simulink model "HvacMdlAirFlowDistbnFrnt"  (R2022b)
settings: Solver=FixedStepDiscrete  StartTime=0.0  StopTime=10.0  FixedStep=0.2  RelTol=1e-3
(part 1 of 2)
settings Model: Creator=blue.ge  LastModifiedBy=blue.ge
settings DataIOCC: SaveFormat=StructureWithTime
settings OptimizationCC: DefaultParameterBehavior=Inlined  EnhancedBackFolding=on  PassReuseOutputArgsAs=Individual arguments  ZeroExternalMemoryAtStartup=off  ZeroInternalMemoryAtStartup=off  OptimizeBlockOrder=speed  DifferentSizesBufferReuse=on  OptimizationCustomize=off  EfficientTunableParamExpr=on
settings DebuggingCC: ParameterTunabilityLossMsg=error
settings ModelReferenceCC: ModelReferenceNumInstancesAllowed=Single
settings SFSimCC: MATLABDynamicMemAlloc=off
settings RTWCC: Description=AUTOSAR  SystemTargetFile=autosar.tlc  GenCodeOnly=on  TemplateMakefile=ert_default_tmf
settings CodeAppCC: ForceParamTrailComments=on  IgnoreCustomStorageClasses=off  OperatorAnnotations=on  SimulinkDataObjDesc=on  SFDataObjDesc=on  InsertBlockDesc=on
settings STFCustomTargetCC: TargetLangStandard=C99 (ISO)  MultiwordLength=256  ParMdlRefBuildCompliant=on  CompOptLevelCompliant=on  IncludeMdlTerminateFcn=off  CombineOutputUpdateFcns=on  SuppressErrorStatus=on  ERTCustomFileBanners=on  SupportNonFinite=off  SupportComplex=off  SupportContinuousTime=off  SupportNonInlinedSFcns=off  RemoveResetFunc=on  MATLABClassNameForMDSCustomization=Simulink.SoftwareTarget.ERTCustomization  AutosarCompliant=on  UseToolchainInfoCompliant=on  LookupTableObjectStructAxisOrder=2,1,3,4,...  LUTObjectStructOrderEvenSpacing=Size,Table,Breakpoints  SystemTargetFile=autosar.tlc
settings ERTTargetCC: TargetLangStandard=C99 (ISO)  ConcurrentExecutionCompliant=off  IncludeMdlTerminateFcn=off  SuppressErrorStatus=on  SupportNonFinite=off  SupportComplex=off  ModelStepFunctionPrototypeControlCompliant=off  CPPClassGenCompliant=off  AutosarCompliant=on  LookupTableObjectStructAxisOrder=2,1,3,4,...  LUTObjectStructOrderEvenSpacing=Size,Table,Breakpoints  InlinedParameterPlacement=NonHierarchical  ERTCustomFileTemplate=example_file_process.tlc

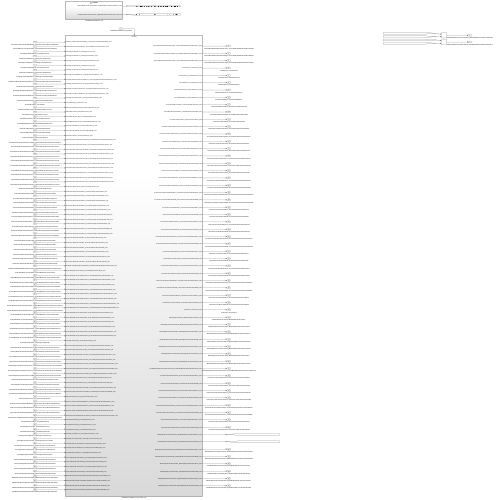
[thumbnail: Simulink's stored preview of the top-level diagram]
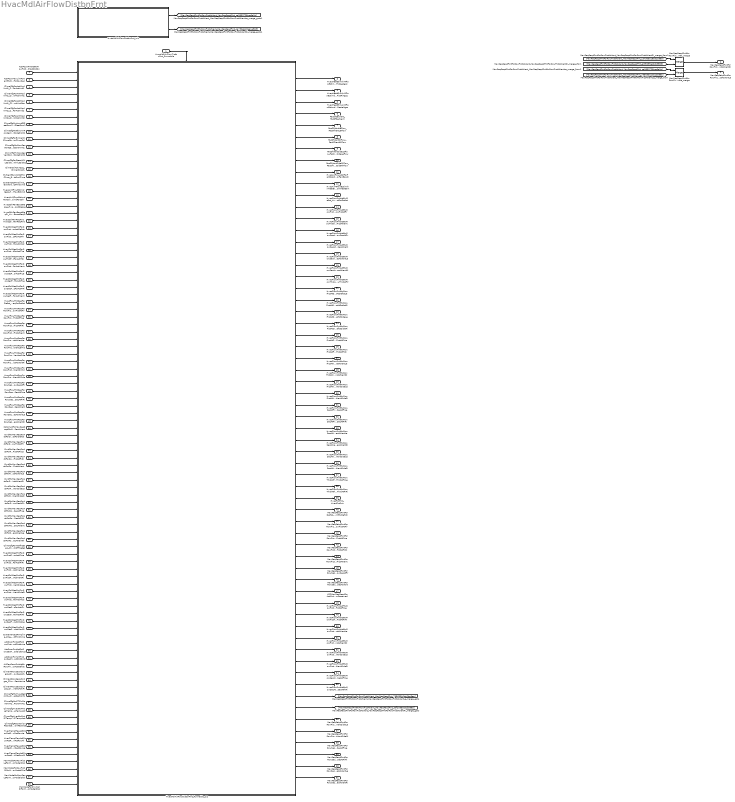
== system HvacMdlAirFlowDistbnFrnt ==
  HvacMdlAirFlowDistbnFrnt_Runnable : Inport  SampleTime=0.2  OutputFunctionCall=on
  ActrPosnFrntActForRowFirstModDistbn_ActrPosnFrntActForRowFirstModDistbn : Inport  Port=2  OutMin=0  OutMax=100  OutDataTypeStr=Perc9  PortDimensions=1
  ActrPosnMaxLimForVentForRowSec_ActrPosnMaxLimForVentForRowSec : Inport  Port=3  OutDataTypeStr=Perc30  PortDimensions=1
  ClimaCfgForActrModVrnt1_ClimaCfgForActrInd1 : Inport  Port=4  OutDataTypeStr=Enum: ClimaCfgForActrInd1  PortDimensions=1
  ClimaCfgForActrModVrnt1_ClimaCfgForActrModVrnt1 : Inport  Port=5  OutDataTypeStr=Enum: ClimaCfgForActrModVrnt1  PortDimensions=1
  ClimaCfgForActrModVrnt1_ClimaCfgForActrModVrntCen : Inport  Port=6  OutDataTypeStr=Enum: ClimaCfgForActrModVrntCen  PortDimensions=1
  ClimaCfgForActrModVrnt2_ClimaCfgForActrInd2 : Inport  Port=7  OutDataTypeStr=Enum: ClimaCfgForActrInd2  PortDimensions=1
  ClimaCfgForActrModVrnt2_ClimaCfgForActrModVrnt2 : Inport  Port=8  OutDataTypeStr=Enum: ClimaCfgForActrModVrnt2  PortDimensions=1
  ClimaCfgForAmntOfSeatRow1_ClimaCfgForAmntOfSeatRow1 : Inport  Port=9  OutDataTypeStr=Enum: ClimaCfgForAmntOfSeatRow1  PortDimensions=1
  ClimaCfgForBlowrInRowSecVentSidePrsnt1_ClimaCfgForBlowrInRowSecVentSidePrsnt1 : Inport  Port=10  OutDataTypeStr=Boolean  PortDimensions=1
  ClimaCfgForExtVentnClimaCtrl_ClimaCfgForExtVentnClimaCtrl : Inport  Port=11  OutDataTypeStr=Enum: CarCfgForExtVentnClimaCtrl  PortDimensions=1
  ClimaCfgForRowSecVentCentrVrnt1_ClimaCfgForRowSecVentCentrVrnt1 : Inport  Port=12  OutDataTypeStr=Enum: ClimaCfgForRowSecVentCentrVrnt1  PortDimensions=1
  ClimaCfgForRowSecVentSidePrsnt1_ClimaCfgForRowSecVentSidePrsnt1 : Inport  Port=13  OutDataTypeStr=Boolean  PortDimensions=1
  ClimaCfgForSteerWhlLeSide1_ClimaCfgForSteerWhlLeSide1 : Inport  Port=14  OutDataTypeStr=Boolean  PortDimensions=1
  ClimaCtrlActvNgcc_ClimaCtrlActv : Inport  Port=15  OutDataTypeStr=Boolean  PortDimensions=1
  ExtVentFanVolStsFrmClima_ExtVentFanVolStsFrmClima : Inport  Port=16  OutDataTypeStr=Enum: Level4  PortDimensions=1
  ExtVentStsFrmClima_ExtVentStsFrmClima : Inport  Port=17  OutDataTypeStr=Enum: OnOff1  PortDimensions=1
  HvacAirMFlowEstimd_Ngcc_HvacAirMFlowEstimd : Inport  Port=18  OutMin=0  OutMax=1000  OutDataTypeStr=MFlow1  PortDimensions=1
  HvacAirMFlowEstimdForCtrlr_HvacAirMFlowEstimdForCtrlr : Inport  Port=19  OutDataTypeStr=AirFlow30  PortDimensions=1
  HvacCtrlrFanBoostReqLe_HvacCtrlrFanBoostReqLe : Inport  Port=20  OutDataTypeStr=Perc30  PortDimensions=1
  HvacCtrlrFanBoostReqRi_HvacCtrlrFanBoostReqRi : Inport  Port=21  OutDataTypeStr=Perc30  PortDimensions=1
  HvacCtrlrFanReqFrnt_HvacCtrlrFanReqFrnt : Inport  Port=22  OutDataTypeStr=FanSpd1  PortDimensions=1
  HvacCtrlrReqFrntForRowFirstForActrDefrst_HvacCtrlrReqFrntForRowFirstForActrDefrst : Inport  Port=23  OutDataTypeStr=Perc30  PortDimensions=1
  HvacCtrlrReqFrntForRowFirstForActrFlr_HvacCtrlrReqFrntForRowFirstForActrFlr : Inport  Port=24  OutDataTypeStr=Perc30  PortDimensions=1
  HvacCtrlrReqFrntForRowFirstForActrMod_HvacCtrlrReqFrntForRowFirstForActrMod : Inport  Port=25  OutDataTypeStr=Perc30  PortDimensions=1
  HvacCtrlrReqFrntForRowFirstForActrTLe_HvacCtrlrReqFrntForRowFirstForActrTLe : Inport  Port=26  OutDataTypeStr=Perc30  PortDimensions=1
  HvacCtrlrReqFrntForRowFirstForActrTRi_HvacCtrlrReqFrntForRowFirstForActrTRi : Inport  Port=27  OutDataTypeStr=Perc30  PortDimensions=1
  HvacCtrlrReqFrntForRowFirstForActrVent_HvacCtrlrReqFrntForRowFirstForActrVent : Inport  Port=28  OutDataTypeStr=Perc30  PortDimensions=1
  HvacCtrlrReqFrntForRowSecForActrFlr_HvacCtrlrReqFrntForRowSecForActrFlrLe : Inport  Port=29  OutDataTypeStr=Perc30  PortDimensions=1
  HvacCtrlrReqFrntForRowSecForActrTLe_HvacCtrlrReqFrntForRowSecForActrTLe : Inport  Port=30  OutDataTypeStr=Perc30  PortDimensions=1
  HvacCtrlrReqFrntForRowSecForActrTRi_HvacCtrlrReqFrntForRowSecForActrTRi : Inport  Port=31  OutDataTypeStr=Perc30  PortDimensions=1
  HvacCtrlrReqFrntForRowSecForActrVent_HvacCtrlrReqFrntForRowSecForActrVent : Inport  Port=32  OutDataTypeStr=Perc30  PortDimensions=1
  HvacFlowFrntReqForDefrst_HvacFlowFrntReqForDefrst : Inport  Port=33  OutDataTypeStr=AirFlow30  PortDimensions=1
  HvacFlowFrntReqForRowFirstAtFlr_HvacFlowFrntReqForRowFirstAtFlr : Inport  Port=34  OutDataTypeStr=AirFlow30  PortDimensions=1
  HvacFlowFrntReqForRowFirstAtFlr_HvacFlowFrntReqForRowFirstAtFlrLe : Inport  Port=35  OutDataTypeStr=AirFlow30  PortDimensions=1
  HvacFlowFrntReqForRowFirstAtFlr_HvacFlowFrntReqForRowFirstAtFlrRi : Inport  Port=36  OutDataTypeStr=AirFlow30  PortDimensions=1
  HvacFlowFrntReqForRowFirstAtVent_HvacFlowFrntReqForRowFirstAtVent : Inport  Port=37  OutDataTypeStr=AirFlow30  PortDimensions=1
  HvacFlowFrntReqForRowFirstAtVent_HvacFlowFrntReqForRowFirstAtVentLe : Inport  Port=38  OutDataTypeStr=AirFlow30  PortDimensions=1
  HvacFlowFrntReqForRowFirstAtVent_HvacFlowFrntReqForRowFirstAtVentLeMid : Inport  Port=39  OutDataTypeStr=AirFlow30  PortDimensions=1
  HvacFlowFrntReqForRowFirstAtVent_HvacFlowFrntReqForRowFirstAtVentLeSide : Inport  Port=40  OutDataTypeStr=AirFlow30  PortDimensions=1
  HvacFlowFrntReqForRowFirstAtVent_HvacFlowFrntReqForRowFirstAtVentRi : Inport  Port=41  OutDataTypeStr=AirFlow30  PortDimensions=1
  HvacFlowFrntReqForRowFirstAtVent_HvacFlowFrntReqForRowFirstAtVentRiMid : Inport  Port=42  OutDataTypeStr=AirFlow30  PortDimensions=1
  HvacFlowFrntReqForRowFirstAtVent_HvacFlowFrntReqForRowFirstAtVentRiSide : Inport  Port=43  OutDataTypeStr=AirFlow30  PortDimensions=1
  HvacFlowFrntReqForRowSecAtFlr_HvacFlowFrntReqForRowSecAtFlr : Inport  Port=44  OutDataTypeStr=AirFlow30  PortDimensions=1
  HvacFlowFrntReqForRowSecAtFlr_HvacFlowFrntReqForRowSecAtFlrLe : Inport  Port=45  OutDataTypeStr=AirFlow30  PortDimensions=1
  HvacFlowFrntReqForRowSecAtFlr_HvacFlowFrntReqForRowSecAtFlrRi : Inport  Port=46  OutDataTypeStr=AirFlow30  PortDimensions=1
  HvacFlowFrntReqForRowSecAtVent_HvacFlowFrntReqForRowSecAtVent : Inport  Port=47  OutDataTypeStr=AirFlow30  PortDimensions=1
  HvacFlowFrntReqForRowSecAtVent_HvacFlowFrntReqForRowSecAtVentLe : Inport  Port=48  OutDataTypeStr=AirFlow30  PortDimensions=1
  HvacFlowFrntReqForRowSecAtVent_HvacFlowFrntReqForRowSecAtVentRi : Inport  Port=49  OutDataTypeStr=AirFlow30  PortDimensions=1
  NotAllwdForMaxReqReqdForRowSecAtVent_NotAllwdForMaxReqReqdForRowSecAtVent : Inport  Port=50  OutDataTypeStr=Boolean  PortDimensions=1
  OvrdForMaxReqForActrForDefrst_OvrdForMaxReqForActrForDefrst : Inport  Port=51  OutDataTypeStr=Boolean  PortDimensions=1
  OvrdForMaxReqForActrForRowFirstAtFlr_OvrdForMaxReqForActrForRowFirstAtFlr : Inport  Port=52  OutDataTypeStr=Boolean  PortDimensions=1
  OvrdForMaxReqForActrForRowFirstAtFlr_OvrdForMaxReqForActrForRowFirstAtFlrLe : Inport  Port=53  OutDataTypeStr=Boolean  PortDimensions=1
  OvrdForMaxReqForActrForRowFirstAtFlr_OvrdForMaxReqForActrForRowFirstAtFlrRi : Inport  Port=54  OutDataTypeStr=Boolean  PortDimensions=1
  OvrdForMaxReqForActrForRowFirstAtVent_OvrdForMaxReqForActrForRowFirstAtVent : Inport  Port=55  OutDataTypeStr=Boolean  PortDimensions=1
  OvrdForMaxReqForActrForRowFirstAtVent_OvrdForMaxReqForActrForRowFirstAtVentLe : Inport  Port=56  OutDataTypeStr=Boolean  PortDimensions=1
  OvrdForMaxReqForActrForRowFirstAtVent_OvrdForMaxReqForActrForRowFirstAtVentRi : Inport  Port=57  OutDataTypeStr=Boolean  PortDimensions=1
  OvrdForMaxReqForActrForRowFirstAtVent_OvrdForMaxReqForActrForRowFirstAtVentSideLe : Inport  Port=58  OutDataTypeStr=Boolean  PortDimensions=1
  OvrdForMaxReqForActrForRowFirstAtVent_OvrdForMaxReqForActrForRowFirstAtVentSideRi : Inport  Port=59  OutDataTypeStr=Boolean  PortDimensions=1
  OvrdForMaxReqForActrForRowSecAtFlr_OvrdForMaxReqForActrForRowSecAtFlr : Inport  Port=60  OutDataTypeStr=Boolean  PortDimensions=1
  OvrdForMaxReqForActrForRowSecAtFlr_OvrdForMaxReqForActrForRowSecAtFlrLe : Inport  Port=61  OutDataTypeStr=Boolean  PortDimensions=1
  OvrdForMaxReqForActrForRowSecAtFlr_OvrdForMaxReqForActrForRowSecAtFlrRi : Inport  Port=62  OutDataTypeStr=Boolean  PortDimensions=1
  OvrdForMaxReqForActrForRowSecAtVent_OvrdForMaxReqForActrForRowSecAtVent : Inport  Port=63  OutDataTypeStr=Boolean  PortDimensions=1
  OvrdForMaxReqForActrForRowSecAtVent_OvrdForMaxReqForActrForRowSecAtVentLe : Inport  Port=64  OutDataTypeStr=Boolean  PortDimensions=1
  OvrdForMaxReqForActrForRowSecAtVent_OvrdForMaxReqForActrForRowSecAtVentRi : Inport  Port=65  OutDataTypeStr=Boolean  PortDimensions=1
  ClimaCfgForNrOfTSetg1_ClimaCfgForNrOfTSetg1 : Inport  Port=66  OutDataTypeStr=Enum: ClimaCfgForNrOfTSetg1  PortDimensions=1
  HvacCtrlrReqFrntForRowFirstForActrFlr_HvacCtrlrReqFrntForRowFirstForActrFlrLe : Inport  Port=67  OutDataTypeStr=Perc30  PortDimensions=1
  HvacCtrlrReqFrntForRowFirstForActrFlr_HvacCtrlrReqFrntForRowFirstForActrFlrRi : Inport  Port=68  OutDataTypeStr=Perc30  PortDimensions=1
  HvacCtrlrReqFrntForRowFirstForActrVent_HvacCtrlrReqFrntForRowFirstForActrVentLe : Inport  Port=69  OutDataTypeStr=Perc30  PortDimensions=1
  HvacCtrlrReqFrntForRowFirstForActrVent_HvacCtrlrReqFrntForRowFirstForActrVentRi : Inport  Port=70  OutDataTypeStr=Perc30  PortDimensions=1
  HvacCtrlrReqFrntForRowFirstForActrVent_HvacCtrlrReqFrntForRowFirstForActrVentSideLe : Inport  Port=71  OutDataTypeStr=Perc30  PortDimensions=1
  HvacCtrlrReqFrntForRowFirstForActrVent_HvacCtrlrReqFrntForRowFirstForActrVentSideRi : Inport  Port=72  OutDataTypeStr=Perc30  PortDimensions=1
  HvacCtrlrReqFrntForRowFirstLeForActrMod_HvacCtrlrReqFrntForRowFirstLeForActrMod : Inport  Port=73  OutDataTypeStr=Perc30  PortDimensions=1
  HvacCtrlrReqFrntForRowSecForActrFlr_HvacCtrlrReqFrntForRowSecForActrFlr : Inport  Port=74  OutDataTypeStr=Perc30  PortDimensions=1
  HvacCtrlrReqFrntForRowSecForActrFlr_HvacCtrlrReqFrntForRowSecForActrFlrRi : Inport  Port=75  OutDataTypeStr=Perc30  PortDimensions=1
  HvacCtrlrReqFrntForRowSecForActrModLe_HvacCtrlrReqFrntForRowSecForActrModLe : Inport  Port=76  OutDataTypeStr=Perc30  PortDimensions=1
  HvacCtrlrReqFrntForRowSecForActrModRi_HvacCtrlrReqFrntForRowSecForActrModRi : Inport  Port=77  OutDataTypeStr=Perc30  PortDimensions=1
  ExtVentToastFrmClima_Prkg_ExtVentToastFrmClima : Inport  Port=78  OutDataTypeStr=Enum: ExtVentToastFrmClimaSts  PortDimensions=1
  ActrPosnFrntActForRowFirstModDistbnLe_ActrPosnFrntActForRowFirstModDistbnLe : Inport  Port=79  OutMin=0  OutMax=100  OutDataTypeStr=Perc9  PortDimensions=1
  ActrPosnFrntActForRowSecModDistbnLe_ActrPosnFrntActForRowSecModDistbnLe : Inport  Port=80  OutMin=0  OutMax=100  OutDataTypeStr=Perc9  PortDimensions=1
  ActrPosnFrntActForRowSecModDistbnRi_ActrPosnFrntActForRowSecModDistbnRi : Inport  Port=81  OutMin=0  OutMax=100  OutDataTypeStr=Perc9  PortDimensions=1
  AirFlapPosnFrntActForRowFirstForActrDefrst_AirFlapPosnFrntActForRowFirstForActrDefrst : Inport  Port=82  OutMin=0  OutMax=100  OutDataTypeStr=Perc9  PortDimensions=1
  ClimaAtRowSecActvNgcc_ClimaAtRowSecActv : Inport  Port=83  OutDataTypeStr=Boolean  PortDimensions=1
  ClimaAtRowSecActvNgcc_ClimaAtRowSecActvLe : Inport  Port=84  OutDataTypeStr=Boolean  PortDimensions=1
  ClimaAtRowSecActvNgcc_ClimaAtRowSecActvRi : Inport  Port=85  OutDataTypeStr=Boolean  PortDimensions=1
  ClimaCfgForHvacRePrsnt1_ClimaCfgForHvacRePrsnt1 : Inport  Port=86  OutDataTypeStr=Enum: CarCfgForHvacRe  PortDimensions=1
  ClimaCfgForPTCInFrontHVAC_ClimaCfgForPTCInFrontHVAC : Inport  Port=87  OutDataTypeStr=Enum: FrontHeaterExchanger  PortDimensions=1
  ClimaCfgHvacForFrntLeTempActr_ClimaCfgHvacForFrntLeTempActr : Inport  Port=88  OutDataTypeStr=Boolean  PortDimensions=1
  ClimaCfgHvacForFrntRiTempActr_ClimaCfgHvacForFrntRiTempActr : Inport  Port=89  OutDataTypeStr=Boolean  PortDimensions=1
  ClimaCfgReqVersionReplace_ClimaCfgReqVersionReplace : Inport  Port=90  OutDataTypeStr=UInt8  PortDimensions=1
  HvacTempFlapActlPosnFrstRowLe_HvacTempFlapActlPosnFrstRowLe : Inport  Port=91  OutMin=0  OutMax=101  OutDataTypeStr=Perc101  PortDimensions=1
  HvacTempFlapActlPosnFrstRowRi_HvacTempFlapActlPosnFrstRowRi : Inport  Port=92  OutMin=0  OutMax=101  OutDataTypeStr=Perc101  PortDimensions=1
  HvacTempFlapActlPosnSecRowLe_HvacTempFlapActlPosnSecRowLe : Inport  Port=93  OutMin=0  OutMax=101  OutDataTypeStr=Perc101  PortDimensions=1
  HvacTempFlapActlPosnSecRowRi_HvacTempFlapActlPosnSecRowRi : Inport  Port=94  OutMin=0  OutMax=101  OutDataTypeStr=Perc101  PortDimensions=1
  ManModeForRowFirstLeForModelDial_ManModeForRowFirstLeForModelDial : Inport  Port=95  OutDataTypeStr=Enum: HmiCmptmtAirDistbnFrnt  PortDimensions=1
  ManModeForRowFirstRiForModelDial_ManModeForRowFirstRiForModelDial : Inport  Port=96  OutDataTypeStr=Enum: HmiCmptmtAirDistbnFrnt  PortDimensions=1
  ManModeForRowSecLeForModelDial_ManModeForRowSecLeForModelDial : Inport  Port=97  OutDataTypeStr=Enum: HmiCmptmtAirDistbnFrnt  PortDimensions=1
  ManModeForRowSecRiForModelDial_ManModeForRowSecRiForModelDial : Inport  Port=98  OutDataTypeStr=Enum: HmiCmptmtAirDistbnFrnt  PortDimensions=1
  HvacMdlAirFlowDistbnFrnt_Init : Subsystem
  HvacMdlAirFlowDistbnFrnt_Runnable_sys : Subsystem
  MaxReqReqdFrntForRowFirstAtVent_MaxReqReqdFrntForRowFirstAtVentLe_merge : Merge
  MaxReqReqdFrntForRowFirstAtVent_MaxReqReqdFrntForRowFirstAtVentRi_merge : Merge
  MaxReqReqdFrntForRowFirstAtVent_MaxReqReqdFrntForRowFirstAtVentLe : Outport  OutDataTypeStr=Boolean  PortDimensions=1
  MaxReqReqdFrntForRowFirstAtVent_MaxReqReqdFrntForRowFirstAtVentRi : Outport  Port=2  OutDataTypeStr=Boolean  PortDimensions=1
  HvacHeatElmAirMFlowEstimdFirstLeNgcc_HvacHeatElmAirMFlowEstimdFirstLeNgcc : Outport  Port=3  OutDataTypeStr=AirFlow30  PortDimensions=1
  HvacHeatElmAirMFlowEstimdFirstRiNgcc_HvacHeatElmAirMFlowEstimdFirstRiNgcc : Outport  Port=4  OutDataTypeStr=AirFlow30  PortDimensions=1
  HvacHeatElmAirMFlowEstimdSecLeNgcc_HvacHeatElmAirMFlowEstimdSecLeNgcc : Outport  Port=5  OutDataTypeStr=AirFlow30  PortDimensions=1
  FacOfSecVent_FacOfSecVent : Outport  Port=6  OutDataTypeStr=VCCFac30  PortDimensions=1
  FacOfVentLeFlow_FacOfVentLeFlow : Outport  Port=7  OutDataTypeStr=VCCFac30  PortDimensions=1
  FacOfVentRiFlow_FacOfVentRiFlow : Outport  Port=8  OutDataTypeStr=VCCFac30  PortDimensions=1
  FacOfVentSideLeFlow_FacOfVentSideLeFlow : Outport  Port=9  OutDataTypeStr=VCCFac30  PortDimensions=1
  FacOfVentSideRiFlow_FacOfVentSideRiFlow : Outport  Port=10  OutDataTypeStr=VCCFac30  PortDimensions=1
  HvacAirFlowAddlForFanBoost_HvacAirFlowAddlForFanBoost : Outport  Port=11  OutDataTypeStr=AirFlow30  PortDimensions=1
  HvacAirMFlowEstimdInHeatElm_HvacAirMFlowEstimdInHeatElm : Outport  Port=12  OutDataTypeStr=AirFlow30  PortDimensions=1
  HvacFlowFrntActForDefrst_HvacFlowFrntActForDefrst : Outport  Port=13  OutDataTypeStr=AirFlow30  PortDimensions=1
  HvacFlowFrntActForRowFirstAtFlr_HvacFlowFrntActForRowFirstAtFlr : Outport  Port=14  OutDataTypeStr=AirFlow30  PortDimensions=1
  HvacFlowFrntActForRowFirstAtVent_HvacFlowFrntActForRowFirstAtVent : Outport  Port=15  OutDataTypeStr=AirFlow30  PortDimensions=1
  HvacFlowFrntActForRowSecAtFlr_HvacFlowFrntActForRowSecAtFlr : Outport  Port=16  OutDataTypeStr=AirFlow30  PortDimensions=1
  HvacFlowFrntActForRowSecAtVent_HvacFlowFrntActForRowSecAtVent : Outport  Port=17  OutDataTypeStr=AirFlow30  PortDimensions=1
  HvacFlowFrntActForRowSecAtVent_HvacFlowFrntActForRowSecAtVentLe : Outport  Port=18  OutDataTypeStr=AirFlow30  PortDimensions=1
  HvacFlowFrntActForRowSecAtVent_HvacFlowFrntActForRowSecAtVentRi : Outport  Port=19  OutDataTypeStr=AirFlow30  PortDimensions=1
  HvacFlowFrntActForRowThrdAtFlr_HvacFlowFrntActForRowThrdAtFlr : Outport  Port=20  OutDataTypeStr=AirFlow30  PortDimensions=1
  HvacFlowFrntForRowFirstAtDefrstLe_HvacFlowFrntForRowFirstAtDefrstLe : Outport  Port=21  OutDataTypeStr=AirFlow30  PortDimensions=1
  HvacFlowFrntForRowFirstAtDefrstRi_HvacFlowFrntForRowFirstAtDefrstRi : Outport  Port=22  OutDataTypeStr=AirFlow30  PortDimensions=1
  HvacFlowFrntForRowFirstAtDefrstSideLe_HvacFlowFrntForRowFirstAtDefrstSideLe : Outport  Port=23  OutDataTypeStr=AirFlow30  PortDimensions=1
  HvacFlowFrntForRowFirstAtDefrstSideRi_HvacFlowFrntForRowFirstAtDefrstSideRi : Outport  Port=24  OutDataTypeStr=AirFlow30  PortDimensions=1
  HvacFlowFrntForRowFirstAtFlrLe_HvacFlowFrntForRowFirstAtFlrLe : Outport  Port=25  OutDataTypeStr=AirFlow30  PortDimensions=1
  HvacFlowFrntForRowFirstAtFlrRi_HvacFlowFrntForRowFirstAtFlrRi : Outport  Port=26  OutDataTypeStr=AirFlow30  PortDimensions=1
  HvacFlowFrntForRowFirstAtVentLe_HvacFlowFrntForRowFirstAtVentLe : Outport  Port=27  OutDataTypeStr=AirFlow30  PortDimensions=1
  HvacFlowFrntForRowFirstAtVentRi_HvacFlowFrntForRowFirstAtVentRi : Outport  Port=28  OutDataTypeStr=AirFlow30  PortDimensions=1
  HvacFlowFrntForRowFirstAtVentSideLe_HvacFlowFrntForRowFirstAtVentSideLe : Outport  Port=29  OutDataTypeStr=AirFlow30  PortDimensions=1
  HvacFlowFrntForRowFirstAtVentSideRi_HvacFlowFrntForRowFirstAtVentSideRi : Outport  Port=30  OutDataTypeStr=AirFlow30  PortDimensions=1
  HvacFlowFrntForRowSecAtFlrLe_HvacFlowFrntForRowSecAtFlrLe : Outport  Port=31  OutDataTypeStr=AirFlow30  PortDimensions=1
  HvacFlowFrntForRowSecAtFlrRi_HvacFlowFrntForRowSecAtFlrRi : Outport  Port=32  OutDataTypeStr=AirFlow30  PortDimensions=1
  HvacFlowFrntForRowSecAtVentLe_HvacFlowFrntForRowSecAtVentLe : Outport  Port=33  OutDataTypeStr=AirFlow30  PortDimensions=1
  HvacFlowFrntForRowSecAtVentRi_HvacFlowFrntForRowSecAtVentRi : Outport  Port=34  OutDataTypeStr=AirFlow30  PortDimensions=1
  HvacFlowFrntForRowSecAtVentSideLe_HvacFlowFrntForRowSecAtVentSideLe : Outport  Port=35  OutDataTypeStr=AirFlow30  PortDimensions=1
  HvacFlowFrntForRowSecAtVentSideRi_HvacFlowFrntForRowSecAtVentSideRi : Outport  Port=36  OutDataTypeStr=AirFlow30  PortDimensions=1
  HvacFlowFrntForRowThrdAtFlrLe_HvacFlowFrntForRowThrdAtFlrLe : Outport  Port=37  OutDataTypeStr=AirFlow30  PortDimensions=1
  HvacFlowFrntForRowThrdAtFlrRi_HvacFlowFrntForRowThrdAtFlrRi : Outport  Port=38  OutDataTypeStr=AirFlow30  PortDimensions=1
  HvacFlrsFrnt_HvacFlrsFrnt : Outport  Port=39  OutDataTypeStr=Flrs30  PortDimensions=1
  MaxReqReqdFrntForDefrst_MaxReqReqdFrntForDefrst : Outport  Port=40  OutDataTypeStr=Boolean  PortDimensions=1
  MaxReqReqdFrntForRowFirstAtFlr_MaxReqReqdFrntForRowFirstAtFlr : Outport  Port=41  OutDataTypeStr=Boolean  PortDimensions=1
  MaxReqReqdFrntForRowFirstAtFlr_MaxReqReqdFrntForRowFirstAtFlrLe : Outport  Port=42  OutDataTypeStr=Boolean  PortDimensions=1
  MaxReqReqdFrntForRowFirstAtFlr_MaxReqReqdFrntForRowFirstAtFlrRi : Outport  Port=43  OutDataTypeStr=Boolean  PortDimensions=1
  MaxReqReqdFrntForRowFirstAtVent_MaxReqReqdFrntForRowFirstAtVent : Outport  Port=44  OutDataTypeStr=Boolean  PortDimensions=1
  MaxReqReqdFrntForRowSecAtFlr_MaxReqReqdFrntForRowSecAtFlr : Outport  Port=45  OutDataTypeStr=Boolean  PortDimensions=1
  MaxReqReqdFrntForRowSecAtVent_MaxReqReqdFrntForRowSecAtVent : Outport  Port=46  OutDataTypeStr=Boolean  PortDimensions=1
  VCCMaxReqReqdForActrFrntEnumReserved_VCCMaxReqReqdForActrFrntEnumReserved : Outport  Port=47  OutDataTypeStr=Enum: VCCMaxReqReqdForActrFrnt  PortDimensions=1
  HvacFlowFrntActForRowFirstAtFlr_HvacFlowFrntActForRowFirstAtFlrLe : Outport  Port=48  OutDataTypeStr=AirFlow30  PortDimensions=1
  HvacFlowFrntActForRowFirstAtFlr_HvacFlowFrntActForRowFirstAtFlrRi : Outport  Port=49  OutDataTypeStr=AirFlow30  PortDimensions=1
  HvacFlowFrntActForRowFirstAtVent_HvacFlowFrntActForRowFirstAtVentLe : Outport  Port=50  OutDataTypeStr=AirFlow30  PortDimensions=1
  HvacFlowFrntActForRowFirstAtVent_HvacFlowFrntActForRowFirstAtVentRi : Outport  Port=51  OutDataTypeStr=AirFlow30  PortDimensions=1
  HvacFlowFrntActForRowFirstAtVent_HvacFlowFrntActForRowFirstAtVentSideLe : Outport  Port=52  OutDataTypeStr=AirFlow30  PortDimensions=1
  HvacFlowFrntActForRowFirstAtVent_HvacFlowFrntActForRowFirstAtVentSideRi : Outport  Port=53  OutDataTypeStr=AirFlow30  PortDimensions=1
  HvacFlowFrntActForRowSecAtFlr_HvacFlowFrntActForRowSecAtFlrLe : Outport  Port=54  OutDataTypeStr=AirFlow30  PortDimensions=1
  HvacFlowFrntActForRowSecAtFlr_HvacFlowFrntActForRowSecAtFlrRi : Outport  Port=55  OutDataTypeStr=AirFlow30  PortDimensions=1
  MaxReqReqdFrntForRowFirstAtVent_MaxReqReqdFrntForRowFirstAtVentSideLe : Outport  Port=56  OutDataTypeStr=Boolean  PortDimensions=1
  MaxReqReqdFrntForRowFirstAtVent_MaxReqReqdFrntForRowFirstAtVentSideRi : Outport  Port=57  OutDataTypeStr=Boolean  PortDimensions=1
  MaxReqReqdFrntForRowSecAtFlr_MaxReqReqdFrntForRowSecAtFlrLe : Outport  Port=58  OutDataTypeStr=Boolean  PortDimensions=1
  MaxReqReqdFrntForRowSecAtFlr_MaxReqReqdFrntForRowSecAtFlrRi : Outport  Port=59  OutDataTypeStr=Boolean  PortDimensions=1
  MaxReqReqdFrntForRowSecAtVent_MaxReqReqdFrntForRowSecAtVentLe : Outport  Port=60  OutDataTypeStr=Boolean  PortDimensions=1
  MaxReqReqdFrntForRowSecAtVent_MaxReqReqdFrntForRowSecAtVentRi : Outport  Port=61  OutDataTypeStr=Boolean  PortDimensions=1
  wires:
  ActrPosnFrntActForRowFirstModDistbn_ActrPosnFrntActForRowFirstModDistbn/1 -> HvacMdlAirFlowDistbnFrnt_Runnable_sys/1(ActrPosnFrntActForRowFirstModDistbn_ActrPosnFrntActForRowFirstModDistbn_read)
  ActrPosnMaxLimForVentForRowSec_ActrPosnMaxLimForVentForRowSec/1 -> HvacMdlAirFlowDistbnFrnt_Runnable_sys/2(ActrPosnMaxLimForVentForRowSec_ActrPosnMaxLimForVentForRowSec_read)
  ClimaCfgForActrModVrnt1_ClimaCfgForActrInd1/1 -> HvacMdlAirFlowDistbnFrnt_Runnable_sys/3(ClimaCfgForActrModVrnt1_ClimaCfgForActrInd1_read)
  ClimaCfgForActrModVrnt1_ClimaCfgForActrModVrnt1/1 -> HvacMdlAirFlowDistbnFrnt_Runnable_sys/4(ClimaCfgForActrModVrnt1_ClimaCfgForActrModVrnt1_read)
  ClimaCfgForActrModVrnt1_ClimaCfgForActrModVrntCen/1 -> HvacMdlAirFlowDistbnFrnt_Runnable_sys/5(ClimaCfgForActrModVrnt1_ClimaCfgForActrModVrntCen_read)
  ClimaCfgForActrModVrnt2_ClimaCfgForActrInd2/1 -> HvacMdlAirFlowDistbnFrnt_Runnable_sys/6(ClimaCfgForActrModVrnt2_ClimaCfgForActrInd2_read)
  ClimaCfgForActrModVrnt2_ClimaCfgForActrModVrnt2/1 -> HvacMdlAirFlowDistbnFrnt_Runnable_sys/7(ClimaCfgForActrModVrnt2_ClimaCfgForActrModVrnt2_read)
  ClimaCfgForAmntOfSeatRow1_ClimaCfgForAmntOfSeatRow1/1 -> HvacMdlAirFlowDistbnFrnt_Runnable_sys/8(ClimaCfgForAmntOfSeatRow1_ClimaCfgForAmntOfSeatRow1_read)
  ClimaCfgForBlowrInRowSecVentSidePrsnt1_ClimaCfgForBlowrInRowSecVentSidePrsnt1/1 -> HvacMdlAirFlowDistbnFrnt_Runnable_sys/9(ClimaCfgForBlowrInRowSecVentSidePrsnt1_ClimaCfgForBlowrInRowSecVentSidePrsnt1_read)
  ClimaCfgForExtVentnClimaCtrl_ClimaCfgForExtVentnClimaCtrl/1 -> HvacMdlAirFlowDistbnFrnt_Runnable_sys/10(ClimaCfgForExtVentnClimaCtrl_ClimaCfgForExtVentnClimaCtrl_read)
  ClimaCfgForRowSecVentCentrVrnt1_ClimaCfgForRowSecVentCentrVrnt1/1 -> HvacMdlAirFlowDistbnFrnt_Runnable_sys/11(ClimaCfgForRowSecVentCentrVrnt1_ClimaCfgForRowSecVentCentrVrnt1_read)
  ClimaCfgForRowSecVentSidePrsnt1_ClimaCfgForRowSecVentSidePrsnt1/1 -> HvacMdlAirFlowDistbnFrnt_Runnable_sys/12(ClimaCfgForRowSecVentSidePrsnt1_ClimaCfgForRowSecVentSidePrsnt1_read)
  ClimaCfgForSteerWhlLeSide1_ClimaCfgForSteerWhlLeSide1/1 -> HvacMdlAirFlowDistbnFrnt_Runnable_sys/13(ClimaCfgForSteerWhlLeSide1_ClimaCfgForSteerWhlLeSide1_read)
  ClimaCtrlActvNgcc_ClimaCtrlActv/1 -> HvacMdlAirFlowDistbnFrnt_Runnable_sys/14(ClimaCtrlActvNgcc_ClimaCtrlActv_read)
  ExtVentFanVolStsFrmClima_ExtVentFanVolStsFrmClima/1 -> HvacMdlAirFlowDistbnFrnt_Runnable_sys/15(ExtVentFanVolStsFrmClima_ExtVentFanVolStsFrmClima_read)
  ExtVentStsFrmClima_ExtVentStsFrmClima/1 -> HvacMdlAirFlowDistbnFrnt_Runnable_sys/16(ExtVentStsFrmClima_ExtVentStsFrmClima_read)
  HvacAirMFlowEstimd_Ngcc_HvacAirMFlowEstimd/1 -> HvacMdlAirFlowDistbnFrnt_Runnable_sys/17(HvacAirMFlowEstimd_Ngcc_HvacAirMFlowEstimd_read)